simulink model "TosAeb"  (R2021a)
settings: Solver=FixedStepDiscrete  StartTime=0.0  StopTime=inf  FixedStep=0.01  RelTol=1e-3
undefined symbols (not defined in the file or in its folder): ACCModSts, AccSts1, AEB_ObjectMovingOrientation, AEB_ObjectMovingState, CN_BTCActive, CN_CurrentGearR, CN_CurrentGearR_DHT, CN_PTCActive, CN_VDCActive, CN_VSC_EspOff, CN_VSC_FCW_AEB_Error, CN_VSC_FCW_AEB_NoDispay, CN_VSC_FCW_AEB_PerfmDgdtn, CN_VSC_FCW_AEB_TempUnAvlbl, CN_VSC_VehStandstill, CNaccelAvoidMax, CNaccelAvoidMin, CNbrakePedalPress, CNcomfortStrLeftAccelMax, CNcomfortStrLeftAccelMin, CNcomfortStrRightAccelMax, CNcomfortStrRightAccelMin, CNDrvDoorOpen, CNDrvModeEco, CNDrvModeNorm, CNDrvModeOther, CNDrvModeSport, CNDrvSBRreminder, CNNull, CNobjInvalidID, CNPassDoorOpen, CNPassSBRreminder, CNPEBSAccelNegInf, CNPEBSAccelNull, CNPEBSTimeInf, CNPEBSTimeNull, CNSTOPMAX, CNSTOPNULL, CNsubjWidth, CNSwtOFF, CNSwtON, CNTTCMAX, CNTTCNULL, CNVehConfigAEBCycNone, CNVehConfigAEBNone, CNVehConfigAEBPedNone, CNVehConfigFCWNone, CNVehConfigJANone, CollisionSeverity, FALSE, FCWSnvty, JACycObjCalcInfoDefaultParams, JAPedObjCalcInfoDefaultParams, JAVehObjCalcInfoDefaultParams, latCycObjCalcInfoDefaultParams, latPedObjCalcInfoDefaultParams, longObjCalcInfoDefaultParams, ModSts, movingStatus, ObjectType ... (+1112)
libraries: simulink x3031, AEBSFunctionLib x50, Ta_Library x33, AEBSStateMachineLib x23, Mylibrary x12, simulink_extras x7, ObjSelect_lib x3, dspstat3 x2
(part 77 of 102)

== system TosAeb/FcwAebJa_Step_sys/ALG_AEBSystem/ALG_AEBController/AEB_JaObjCalcApp/JAVehTgtInfoCalc/AEBJAVehObjCalc/JAVehObjInfoCalc/ObjCrossFlgCal/CollisionProbCalc/Subsystem/ObjBoundingBoxfront/ObjRUCoordinate ==
  inputSignals : Inport
  Add : Sum  Inputs=+--  OutDataTypeStr=single
  Add1 : Sum  Inputs=+-+  OutDataTypeStr=single
  Bus Selector : BusSelector  OutputSignals=dxPreRefPointObj,ObjLengthCorr,sincosCoef.cosCoef,ObjWidthCorr,sincosCoef.sinCoef
  Bus Selector1 : BusSelector  OutputSignals=dyPreRefPointObj,ObjLengthCorr,sincosCoef.sinCoef,ObjWidthCorr,sincosCoef.cosCoef
  Gain : Gain  Gain=0.5  OutDataTypeStr=single
  Gain1 : Gain  Gain=0.5  OutDataTypeStr=single
  Product{,1..3} (4 alike) : Product  OutDataTypeStr=single
  dxPreRUObj : Outport
  dyPreRUObj : Outport  Port=2
  wires:
  inputSignals/1 -> Bus Selector1/1, Bus Selector/1
  Bus Selector/2 -> Product/1  "<ObjLengthCorr>"
  Bus Selector/3 -> Product/2  "<cosCoef>"
  Bus Selector/4 -> Product1/1  "<ObjWidthCorr>"
  Bus Selector/5 -> Product1/2  "<sinCoef>"
  Product1/1 -> Gain1/1
  Bus Selector/1 -> Add/1  "<dxPreRefPointObj>"
  Product/1 -> Add/2
  Add/1 -> dxPreRUObj/1
  Add1/1 -> dyPreRUObj/1
  Product2/1 -> Add1/2
  Product3/1 -> Gain/1
  Bus Selector1/2 -> Product2/1  "<ObjLengthCorr>"
  Bus Selector1/4 -> Product3/1  "<ObjWidthCorr>"
  Bus Selector1/3 -> Product2/2  "<sinCoef>"
  Bus Selector1/5 -> Product3/2  "<cosCoef>"
  Bus Selector1/1 -> Add1/1  "<dyPreRefPointObj>"
  Gain/1 -> Add1/3
  Gain1/1 -> Add/3

== system TosAeb/FcwAebJa_Step_sys/ALG_AEBSystem/ALG_AEBController/AEB_JaObjCalcApp/JAVehTgtInfoCalc/AEBJAVehObjCalc/JAVehObjInfoCalc/ObjCrossFlgCal/CollisionProbCalc/Subsystem/PreCrossFlgCalcFlow/VectorCrossAlgorithm/If Action Subsystem ==
  Action Port : ActionPort  ActionPortLabel=if(u1)
  Constant : Constant  Value=0  OutDataTypeStr=boolean
  CrossFlg : Outport
  wires:
  Constant/1 -> CrossFlg/1

== system TosAeb/FcwAebJa_Step_sys/ALG_AEBSystem/ALG_AEBController/AEB_JaObjCalcApp/JAVehTgtInfoCalc/AEBJAVehObjCalc/JAVehObjInfoCalc/ObjCrossFlgCal/CollisionProbCalc/Subsystem/PreCrossFlgCalcFlow/VectorCrossAlgorithm/If Action Subsystem1 ==
  vectorMulit : Inport
  Coordinations : Inport  Port=2
  Action Port : ActionPort  ActionPortLabel=else
  Bus Selector : BusSelector  OutputSignals=m2,m1,y1,n1,n2,x1
  Bus Selector1 : BusSelector  OutputSignals=x2,x1,y1,n1,y2,m1
  Subsystem : Subsystem
  t : Subsystem
  u : Subsystem
  CrossFlg : Outport
  wires:
  Coordinations/1 -> Bus Selector1/1, Bus Selector/1
  Bus Selector/2 -> t/2(m1)  "<m1>"
  Bus Selector/3 -> t/3(y1)  "<y1>"
  Bus Selector/1 -> t/1(m2)  "<m2>"
  Bus Selector/4 -> t/4(n1)  "<n1>"
  Bus Selector/5 -> t/5(n2)  "<n2>"
  Bus Selector/6 -> t/6(x1)  "<x1>"
  Bus Selector1/3 -> u/3(y1)  "<y1>"
  Bus Selector1/2 -> u/2(x1)  "<x1>"
  Bus Selector1/4 -> u/4(n1)  "<n1>"
  Bus Selector1/1 -> u/1(x2)  "<x2>"
  Bus Selector1/5 -> u/5(y2)  "<y2>"
  Bus Selector1/6 -> u/6(m1)  "<m1>"
  t/1(temp_t) -> Subsystem/1(temp_u)
  u/1(temp_u) -> Subsystem/2(temp_t)
  Subsystem/1(CrossFlg) -> CrossFlg/1
  vectorMulit/1 -> Subsystem/3(vectorMulit)

== system TosAeb/FcwAebJa_Step_sys/ALG_AEBSystem/ALG_AEBController/AEB_JaObjCalcApp/JAVehTgtInfoCalc/AEBJAVehObjCalc/JAVehObjInfoCalc/ObjCrossFlgCal/CollisionProbCalc/Subsystem/PreCrossFlgCalcFlow/VectorCrossAlgorithm/VectorMulit ==
  x1 : Inport  OutDataTypeStr=single
  x2 : Inport  Port=2  OutDataTypeStr=single
  n2 : Inport  Port=3  OutDataTypeStr=single
  n1 : Inport  Port=4  OutDataTypeStr=single
  y2 : Inport  Port=5  OutDataTypeStr=single
  y1 : Inport  Port=6  OutDataTypeStr=single
  m2 : Inport  Port=7  OutDataTypeStr=single
  m1 : Inport  Port=8  OutDataTypeStr=single
  Product : Product  OutDataTypeStr=single
  Product1 : Product  OutDataTypeStr=single
  Subtract{1..4} (4 alike) : Sum  Inputs=+-  OutDataTypeStr=single
  Subtract5 : Sum  Inputs=+-
  vectorMulit : Outport
  wires:
  x1/1 -> Subtract2/2
  x2/1 -> Subtract2/1
  Subtract2/1 -> Product/1
  Subtract3/1 -> Product/2
  y2/1 -> Subtract4/1
  y1/1 -> Subtract4/2
  m2/1 -> Subtract1/1
  m1/1 -> Subtract1/2
  Subtract4/1 -> Product1/1
  Subtract1/1 -> Product1/2
  Product/1 -> Subtract5/1
  Product1/1 -> Subtract5/2
  Subtract5/1 -> vectorMulit/1
  n2/1 -> Subtract3/1
  n1/1 -> Subtract3/2

== system TosAeb/FcwAebJa_Step_sys/ALG_AEBSystem/ALG_AEBController/AEB_JaObjCalcApp/JAVehTgtInfoCalc/AEBJAVehObjCalc/JAVehObjInfoCalc/ObjCrossFlgCal/CollisionProbCalc/Subsystem/SubjBoundingBox/SubjLLCoordinate ==
  inputSignals : Inport
  Add2 : Sum  Inputs=+--  OutDataTypeStr=single
  Add3 : Sum  Inputs=+-+  OutDataTypeStr=single
  Bus Selector2 : BusSelector  OutputSignals=dxPreRefPoint,dxRearBumperToRearAxis,sincosCoef.cosCoef,subjWidth,sincosCoef.sinCoef
  Bus Selector3 : BusSelector  OutputSignals=dyPreRefPoint,dxRearBumperToRearAxis,sincosCoef.sinCoef,subjWidth,sincosCoef.cosCoef
  Gain2 : Gain  Gain=0.5  OutDataTypeStr=single
  Gain3 : Gain  Gain=0.5  OutDataTypeStr=single
  Product{4..7} (4 alike) : Product  OutDataTypeStr=single
  dxPreLL : Outport
  dyPreLL : Outport  Port=2
  wires:
  Gain3/1 -> Add3/3
  Bus Selector3/3 -> Product6/2  "<sinCoef>"
  Add3/1 -> dyPreLL/1
  Product7/1 -> Gain3/1
  Product6/1 -> Add3/2
  Product4/1 -> Add2/2
  Bus Selector3/5 -> Product7/2  "<cosCoef>"
  Product5/1 -> Gain2/1
  Gain2/1 -> Add2/3
  Add2/1 -> dxPreLL/1
  Bus Selector3/4 -> Product7/1  "<subjWidth>"
  Bus Selector3/2 -> Product6/1  "<dxRearBumperToRearAxis>"
  Bus Selector3/1 -> Add3/1  "<dyPreRefPoint>"
  inputSignals/1 -> Bus Selector3/1, Bus Selector2/1
  Bus Selector2/4 -> Product5/1  "<subjWidth>"
  Bus Selector2/2 -> Product4/1  "<dxRearBumperToRearAxis>"
  Bus Selector2/3 -> Product4/2  "<cosCoef>"
  Bus Selector2/1 -> Add2/1  "<dxPreRefPoint>"
  Bus Selector2/5 -> Product5/2  "<sinCoef>"

== system TosAeb/FcwAebJa_Step_sys/ALG_AEBSystem/ALG_AEBController/AEB_JaObjCalcApp/JAVehTgtInfoCalc/AEBJAVehObjCalc/JAVehObjInfoCalc/ObjCrossFlgCal/CollisionProbCalc/Subsystem/SubjBoundingBox/SubjLUCoordinate ==
  inputSignals : Inport
  Add : Sum  Inputs=++-  OutDataTypeStr=single
  Add1 : Sum  Inputs=+++  OutDataTypeStr=single
  Bus Selector : BusSelector  OutputSignals=dxPreRefPoint,dxFrontBumperToRearAxis,sincosCoef.cosCoef,subjWidth,sincosCoef.sinCoef
  Bus Selector1 : BusSelector  OutputSignals=dyPreRefPoint,dxFrontBumperToRearAxis,sincosCoef.sinCoef,subjWidth,sincosCoef.cosCoef
  Gain : Gain  Gain=0.5  OutDataTypeStr=single
  Gain1 : Gain  Gain=0.5  OutDataTypeStr=single
  Product{,1..3} (4 alike) : Product  OutDataTypeStr=single
  dxPreLU : Outport
  dyPreLU : Outport  Port=2
  wires:
  Bus Selector/4 -> Product1/1  "<subjWidth>"
  Product3/1 -> Gain1/1
  Add/1 -> dxPreLU/1
  Bus Selector1/1 -> Add1/1  "<dyPreRefPoint>"
  Gain1/1 -> Add1/3
  Bus Selector1/4 -> Product3/1  "<subjWidth>"
  Product2/1 -> Add1/2
  Bus Selector/3 -> Product/2  "<cosCoef>"
  Add1/1 -> dyPreLU/1
  Bus Selector/5 -> Product1/2  "<sinCoef>"
  Bus Selector/2 -> Product/1  "<dxFrontBumperToRearAxis>"
  Bus Selector1/2 -> Product2/1  "<dxFrontBumperToRearAxis>"
  inputSignals/1 -> Bus Selector1/1, Bus Selector/1
  Bus Selector1/5 -> Product3/2  "<cosCoef>"
  Bus Selector/1 -> Add/1  "<dxPreRefPoint>"
  Gain/1 -> Add/3
  Product1/1 -> Gain/1
  Product/1 -> Add/2
  Bus Selector1/3 -> Product2/2  "<sinCoef>"

== system TosAeb/FcwAebJa_Step_sys/ALG_AEBSystem/ALG_AEBController/AEB_JaObjCalcApp/JAVehTgtInfoCalc/AEBJAVehObjCalc/JAVehObjInfoCalc/ObjCrossFlgCal/CollisionProbCalc/Subsystem/SubjBoundingBox/SubjRLCoordinate ==
  inputSignals : Inport
  Add6 : Sum  Inputs=+-+  OutDataTypeStr=single
  Add7 : Sum  Inputs=+--  OutDataTypeStr=single
  Bus Selector6 : BusSelector  OutputSignals=dxPreRefPoint,dxRearBumperToRearAxis,sincosCoef.cosCoef,subjWidth,sincosCoef.sinCoef
  Bus Selector7 : BusSelector  OutputSignals=dyPreRefPoint,dxRearBumperToRearAxis,sincosCoef.sinCoef,subjWidth,sincosCoef.cosCoef
  Gain6 : Gain  Gain=0.5  OutDataTypeStr=single
  Gain7 : Gain  Gain=0.5  OutDataTypeStr=single
  Product{12..15} (4 alike) : Product  OutDataTypeStr=single
  dxPreRL : Outport
  dyPreRL : Outport  Port=2
  wires:
  Product12/1 -> Add6/2
  Gain6/1 -> Add6/3
  Product13/1 -> Gain6/1
  Add6/1 -> dxPreRL/1
  Bus Selector6/4 -> Product13/1  "<subjWidth>"
  Bus Selector6/5 -> Product13/2  "<sinCoef>"
  Bus Selector6/1 -> Add6/1  "<dxPreRefPoint>"
  Bus Selector6/2 -> Product12/1  "<dxRearBumperToRearAxis>"
  Bus Selector6/3 -> Product12/2  "<cosCoef>"
  inputSignals/1 -> Bus Selector7/1, Bus Selector6/1
  Add7/1 -> dyPreRL/1
  Product14/1 -> Add7/2
  Gain7/1 -> Add7/3
  Bus Selector7/1 -> Add7/1  "<dyPreRefPoint>"
  Bus Selector7/2 -> Product14/1  "<dxRearBumperToRearAxis>"
  Bus Selector7/4 -> Product15/1  "<subjWidth>"
  Product15/1 -> Gain7/1
  Bus Selector7/5 -> Product15/2  "<cosCoef>"
  Bus Selector7/3 -> Product14/2  "<sinCoef>"

== system TosAeb/FcwAebJa_Step_sys/ALG_AEBSystem/ALG_AEBController/AEB_JaObjCalcApp/JAVehTgtInfoCalc/AEBJAVehObjCalc/JAVehObjInfoCalc/ObjCrossFlgCal/CollisionProbCalc/Subsystem/SubjBoundingBox/SubjRUCoordinate ==
  inputSignals : Inport
  Add4 : Sum  Inputs=+++  OutDataTypeStr=single
  Add5 : Sum  Inputs=++-  OutDataTypeStr=single
  Bus Selector4 : BusSelector  OutputSignals=dxPreRefPoint,dxFrontBumperToRearAxis,sincosCoef.cosCoef,subjWidth,sincosCoef.sinCoef
  Bus Selector5 : BusSelector  OutputSignals=dyPreRefPoint,dxFrontBumperToRearAxis,sincosCoef.sinCoef,subjWidth,sincosCoef.cosCoef
  Gain4 : Gain  Gain=0.5  OutDataTypeStr=single
  Gain5 : Gain  Gain=0.5  OutDataTypeStr=single
  Product{8..11} (4 alike) : Product  OutDataTypeStr=single
  dxPreRU : Outport
  dyPreRU : Outport  Port=2
  wires:
  Bus Selector4/4 -> Product9/1  "<subjWidth>"
  Bus Selector4/5 -> Product9/2  "<sinCoef>"
  Bus Selector4/3 -> Product8/2  "<cosCoef>"
  Bus Selector4/1 -> Add4/1  "<dxPreRefPoint>"
  inputSignals/1 -> Bus Selector5/1, Bus Selector4/1
  Bus Selector4/2 -> Product8/1  "<dxFrontBumperToRearAxis>"
  Product9/1 -> Gain4/1
  Bus Selector5/4 -> Product11/1  "<subjWidth>"
  Product10/1 -> Add5/2
  Product11/1 -> Gain5/1
  Add5/1 -> dyPreRU/1
  Gain5/1 -> Add5/3
  Bus Selector5/2 -> Product10/1  "<dxFrontBumperToRearAxis>"
  Bus Selector5/3 -> Product10/2  "<sinCoef>"
  Bus Selector5/5 -> Product11/2  "<cosCoef>"
  Add4/1 -> dxPreRU/1
  Gain4/1 -> Add4/3
  Product8/1 -> Add4/2
  Bus Selector5/1 -> Add5/1  "<dyPreRefPoint>"

== system TosAeb/FcwAebJa_Step_sys/ALG_AEBSystem/ALG_AEBController/AEB_JaObjCalcApp/JaCycTgtInfoCalc/JaCycObjCalcInfoCalc/JaCycObjInfoCalc/ObjCrossFlgCal/CollisionProbCalc/Subsystem/ObjBoundingBox/ObjLLCoordinate ==
  inputSignals : Inport
  Add : Sum  Inputs=+-  OutDataTypeStr=single
  Add1 : Sum  OutDataTypeStr=single
  Bus Selector : BusSelector  OutputSignals=dxPreRefPointObj,ObjWidthCorr,sincosCoef.sinCoef
  Bus Selector1 : BusSelector  OutputSignals=dyPreRefPointObj,ObjWidthCorr,sincosCoef.cosCoef
  Gain : Gain  Gain=0.5  OutDataTypeStr=single
  Gain1 : Gain  Gain=0.5  OutDataTypeStr=single
  Product1 : Product  OutDataTypeStr=single
  Product3 : Product  OutDataTypeStr=single
  dxPre_LLObj : Outport
  dyPreLLObj : Outport  Port=2
  wires:
  Bus Selector1/1 -> Add1/1  "<dyPreRefPointObj>"
  Bus Selector1/3 -> Product3/2  "<cosCoef>"
  Bus Selector1/2 -> Product3/1  "<ObjWidthCorr>"
  Product3/1 -> Gain/1
  Add1/1 -> dyPreLLObj/1
  Add/1 -> dxPre_LLObj/1
  Bus Selector/1 -> Add/1  "<dxPreRefPointObj>"
  Product1/1 -> Gain1/1
  Bus Selector/3 -> Product1/2  "<sinCoef>"
  Bus Selector/2 -> Product1/1  "<ObjWidthCorr>"
  inputSignals/1 -> Bus Selector1/1, Bus Selector/1
  Gain/1 -> Add1/2
  Gain1/1 -> Add/2

== system TosAeb/FcwAebJa_Step_sys/ALG_AEBSystem/ALG_AEBController/AEB_JaObjCalcApp/JaCycTgtInfoCalc/JaCycObjCalcInfoCalc/JaCycObjInfoCalc/ObjCrossFlgCal/CollisionProbCalc/Subsystem/ObjBoundingBox/ObjLUCoordinate ==
  inputSignals : Inport
  Add : Sum  Inputs=++-  OutDataTypeStr=single
  Add1 : Sum  Inputs=+++  OutDataTypeStr=single
  Bus Selector : BusSelector  OutputSignals=dxPreRefPointObj,ObjLengthCorr,sincosCoef.cosCoef,ObjWidthCorr,sincosCoef.sinCoef
  Bus Selector1 : BusSelector  OutputSignals=dyPreRefPointObj,ObjLengthCorr,sincosCoef.sinCoef,ObjWidthCorr,sincosCoef.cosCoef
  Gain : Gain  Gain=0.5  OutDataTypeStr=single
  Gain1 : Gain  Gain=0.5  OutDataTypeStr=single
  Product{,1..3} (4 alike) : Product  OutDataTypeStr=single
  dxPreLUObj : Outport
  dyPreLUobj : Outport  Port=2
  wires:
  inputSignals/1 -> Bus Selector1/1, Bus Selector/1
  Bus Selector/2 -> Product/1  "<ObjLengthCorr>"
  Bus Selector/3 -> Product/2  "<cosCoef>"
  Bus Selector/4 -> Product1/1  "<ObjWidthCorr>"
  Bus Selector/5 -> Product1/2  "<sinCoef>"
  Product1/1 -> Gain1/1
  Bus Selector/1 -> Add/1  "<dxPreRefPointObj>"
  Product/1 -> Add/2
  Add/1 -> dxPreLUObj/1
  Add1/1 -> dyPreLUobj/1
  Product2/1 -> Add1/2
  Product3/1 -> Gain/1
  Bus Selector1/2 -> Product2/1  "<ObjLengthCorr>"
  Bus Selector1/4 -> Product3/1  "<ObjWidthCorr>"
  Bus Selector1/3 -> Product2/2  "<sinCoef>"
  Bus Selector1/5 -> Product3/2  "<cosCoef>"
  Bus Selector1/1 -> Add1/1  "<dyPreRefPointObj>"
  Gain/1 -> Add1/3
  Gain1/1 -> Add/3

== system TosAeb/FcwAebJa_Step_sys/ALG_AEBSystem/ALG_AEBController/AEB_JaObjCalcApp/JaCycTgtInfoCalc/JaCycObjCalcInfoCalc/JaCycObjInfoCalc/ObjCrossFlgCal/CollisionProbCalc/Subsystem/ObjBoundingBox/ObjRLCoordinate ==
  inputSignals : Inport
  Add : Sum  OutDataTypeStr=single
  Add1 : Sum  Inputs=+-  OutDataTypeStr=single
  Bus Selector : BusSelector  OutputSignals=dxPreRefPointObj,ObjWidthCorr,sincosCoef.sinCoef
  Bus Selector1 : BusSelector  OutputSignals=dyPreRefPointObj,ObjWidthCorr,sincosCoef.cosCoef
  Gain : Gain  Gain=0.5  OutDataTypeStr=single
  Gain1 : Gain  Gain=0.5  OutDataTypeStr=single
  Product1 : Product  OutDataTypeStr=single
  Product3 : Product  OutDataTypeStr=single
  dxPreRLObj : Outport
  dyPreRLObj : Outport  Port=2
  wires:
  Bus Selector1/1 -> Add1/1  "<dyPreRefPointObj>"
  Bus Selector1/3 -> Product3/2  "<cosCoef>"
  Bus Selector1/2 -> Product3/1  "<ObjWidthCorr>"
  Product3/1 -> Gain1/1
  Add1/1 -> dyPreRLObj/1
  Add/1 -> dxPreRLObj/1
  Bus Selector/1 -> Add/1  "<dxPreRefPointObj>"
  Product1/1 -> Gain/1
  Bus Selector/3 -> Product1/2  "<sinCoef>"
  Bus Selector/2 -> Product1/1  "<ObjWidthCorr>"
  inputSignals/1 -> Bus Selector1/1, Bus Selector/1
  Gain/1 -> Add/2
  Gain1/1 -> Add1/2

== system TosAeb/FcwAebJa_Step_sys/ALG_AEBSystem/ALG_AEBController/AEB_JaObjCalcApp/JaCycTgtInfoCalc/JaCycObjCalcInfoCalc/JaCycObjInfoCalc/ObjCrossFlgCal/CollisionProbCalc/Subsystem/ObjBoundingBox/ObjRUCoordinate ==
  inputSignals : Inport
  Add : Sum  Inputs=+++  OutDataTypeStr=single
  Add1 : Sum  Inputs=++-  OutDataTypeStr=single
  Bus Selector : BusSelector  OutputSignals=dxPreRefPointObj,ObjLengthCorr,sincosCoef.cosCoef,ObjWidthCorr,sincosCoef.sinCoef
  Bus Selector1 : BusSelector  OutputSignals=dyPreRefPointObj,ObjLengthCorr,sincosCoef.sinCoef,ObjWidthCorr,sincosCoef.cosCoef
  Gain : Gain  Gain=0.5  OutDataTypeStr=single
  Gain1 : Gain  Gain=0.5  OutDataTypeStr=single
  Product{,1..3} (4 alike) : Product  OutDataTypeStr=single
  dxPreRUObj : Outport
  dyPreRUObj : Outport  Port=2
  wires:
  inputSignals/1 -> Bus Selector1/1, Bus Selector/1
  Bus Selector/2 -> Product/1  "<ObjLengthCorr>"
  Bus Selector/3 -> Product/2  "<cosCoef>"
  Bus Selector/4 -> Product1/1  "<ObjWidthCorr>"
  Bus Selector/5 -> Product1/2  "<sinCoef>"
  Product1/1 -> Gain1/1
  Bus Selector/1 -> Add/1  "<dxPreRefPointObj>"
  Product/1 -> Add/2
  Add/1 -> dxPreRUObj/1
  Add1/1 -> dyPreRUObj/1
  Product2/1 -> Add1/2
  Product3/1 -> Gain/1
  Bus Selector1/2 -> Product2/1  "<ObjLengthCorr>"
  Bus Selector1/4 -> Product3/1  "<ObjWidthCorr>"
  Bus Selector1/3 -> Product2/2  "<sinCoef>"
  Bus Selector1/5 -> Product3/2  "<cosCoef>"
  Bus Selector1/1 -> Add1/1  "<dyPreRefPointObj>"
  Gain/1 -> Add1/3
  Gain1/1 -> Add/3

== system TosAeb/FcwAebJa_Step_sys/ALG_AEBSystem/ALG_AEBController/AEB_JaObjCalcApp/JaCycTgtInfoCalc/JaCycObjCalcInfoCalc/JaCycObjInfoCalc/ObjCrossFlgCal/CollisionProbCalc/Subsystem/PreCrossFlgCalcFlow/VectorCrossAlgorithm/If Action Subsystem ==
  Action Port : ActionPort  ActionPortLabel=if(u1)
  Constant : Constant  Value=0  OutDataTypeStr=boolean
  CrossFlg : Outport
  wires:
  Constant/1 -> CrossFlg/1

== system TosAeb/FcwAebJa_Step_sys/ALG_AEBSystem/ALG_AEBController/AEB_JaObjCalcApp/JaCycTgtInfoCalc/JaCycObjCalcInfoCalc/JaCycObjInfoCalc/ObjCrossFlgCal/CollisionProbCalc/Subsystem/PreCrossFlgCalcFlow/VectorCrossAlgorithm/If Action Subsystem1 ==
  vectorMulit : Inport
  Coordinations : Inport  Port=2
  Action Port : ActionPort  ActionPortLabel=else
  Bus Selector : BusSelector  OutputSignals=m2,m1,y1,n1,n2,x1
  Bus Selector1 : BusSelector  OutputSignals=x2,x1,y1,n1,y2,m1
  Subsystem : Subsystem
  t : Subsystem
  u : Subsystem
  CrossFlg : Outport
  wires:
  Coordinations/1 -> Bus Selector1/1, Bus Selector/1
  Bus Selector/2 -> t/2(m1)  "<m1>"
  Bus Selector/3 -> t/3(y1)  "<y1>"
  Bus Selector/1 -> t/1(m2)  "<m2>"
  Bus Selector/4 -> t/4(n1)  "<n1>"
  Bus Selector/5 -> t/5(n2)  "<n2>"
  Bus Selector/6 -> t/6(x1)  "<x1>"
  Bus Selector1/3 -> u/3(y1)  "<y1>"
  Bus Selector1/2 -> u/2(x1)  "<x1>"
  Bus Selector1/4 -> u/4(n1)  "<n1>"
  Bus Selector1/1 -> u/1(x2)  "<x2>"
  Bus Selector1/5 -> u/5(y2)  "<y2>"
  Bus Selector1/6 -> u/6(m1)  "<m1>"
  t/1(temp_t) -> Subsystem/1(temp_u)
  u/1(temp_u) -> Subsystem/2(temp_t)
  Subsystem/1(CrossFlg) -> CrossFlg/1
  vectorMulit/1 -> Subsystem/3(vectorMulit)

== system TosAeb/FcwAebJa_Step_sys/ALG_AEBSystem/ALG_AEBController/AEB_JaObjCalcApp/JaCycTgtInfoCalc/JaCycObjCalcInfoCalc/JaCycObjInfoCalc/ObjCrossFlgCal/CollisionProbCalc/Subsystem/PreCrossFlgCalcFlow/VectorCrossAlgorithm/VectorMulit ==
  x1 : Inport  OutDataTypeStr=single
  x2 : Inport  Port=2  OutDataTypeStr=single
  n2 : Inport  Port=3  OutDataTypeStr=single
  n1 : Inport  Port=4  OutDataTypeStr=single
  y2 : Inport  Port=5  OutDataTypeStr=single
  y1 : Inport  Port=6  OutDataTypeStr=single
  m2 : Inport  Port=7  OutDataTypeStr=single
  m1 : Inport  Port=8  OutDataTypeStr=single
  Product : Product  OutDataTypeStr=single
  Product1 : Product  OutDataTypeStr=single
  Subtract{1..4} (4 alike) : Sum  Inputs=+-  OutDataTypeStr=single
  Subtract5 : Sum  Inputs=+-
  vectorMulit : Outport
  wires:
  x1/1 -> Subtract2/2
  x2/1 -> Subtract2/1
  Subtract2/1 -> Product/1
  Subtract3/1 -> Product/2
  y2/1 -> Subtract4/1
  y1/1 -> Subtract4/2
  m2/1 -> Subtract1/1
  m1/1 -> Subtract1/2
  Subtract4/1 -> Product1/1
  Subtract1/1 -> Product1/2
  Product/1 -> Subtract5/1
  Product1/1 -> Subtract5/2
  Subtract5/1 -> vectorMulit/1
  n2/1 -> Subtract3/1
  n1/1 -> Subtract3/2

== system TosAeb/FcwAebJa_Step_sys/ALG_AEBSystem/ALG_AEBController/AEB_JaObjCalcApp/JaCycTgtInfoCalc/JaCycObjCalcInfoCalc/JaCycObjInfoCalc/ObjCrossFlgCal/CollisionProbCalc/Subsystem/SubjBoundingBox/SubjLLCoordinate ==
  inputSignals : Inport
  Add2 : Sum  Inputs=+--  OutDataTypeStr=single
  Add3 : Sum  Inputs=+-+  OutDataTypeStr=single
  Bus Selector2 : BusSelector  OutputSignals=dxPreRefPoint,dxRearBumperToRearAxis,sincosCoef.cosCoef,subjWidth,sincosCoef.sinCoef
  Bus Selector3 : BusSelector  OutputSignals=dyPreRefPoint,dxRearBumperToRearAxis,sincosCoef.sinCoef,subjWidth,sincosCoef.cosCoef
  Gain2 : Gain  Gain=0.5  OutDataTypeStr=single
  Gain3 : Gain  Gain=0.5  OutDataTypeStr=single
  Product{4..7} (4 alike) : Product  OutDataTypeStr=single
  dxPreLL : Outport
  dyPreLL : Outport  Port=2
  wires:
  Gain3/1 -> Add3/3
  Bus Selector3/3 -> Product6/2  "<sinCoef>"
  Add3/1 -> dyPreLL/1
  Product7/1 -> Gain3/1
  Product6/1 -> Add3/2
  Product4/1 -> Add2/2
  Bus Selector3/5 -> Product7/2  "<cosCoef>"
  Product5/1 -> Gain2/1
  Gain2/1 -> Add2/3
  Add2/1 -> dxPreLL/1
  Bus Selector3/4 -> Product7/1  "<subjWidth>"
  Bus Selector3/2 -> Product6/1  "<dxRearBumperToRearAxis>"
  Bus Selector3/1 -> Add3/1  "<dyPreRefPoint>"
  inputSignals/1 -> Bus Selector3/1, Bus Selector2/1
  Bus Selector2/4 -> Product5/1  "<subjWidth>"
  Bus Selector2/2 -> Product4/1  "<dxRearBumperToRearAxis>"
  Bus Selector2/3 -> Product4/2  "<cosCoef>"
  Bus Selector2/1 -> Add2/1  "<dxPreRefPoint>"
  Bus Selector2/5 -> Product5/2  "<sinCoef>"

== system TosAeb/FcwAebJa_Step_sys/ALG_AEBSystem/ALG_AEBController/AEB_JaObjCalcApp/JaCycTgtInfoCalc/JaCycObjCalcInfoCalc/JaCycObjInfoCalc/ObjCrossFlgCal/CollisionProbCalc/Subsystem/SubjBoundingBox/SubjLUCoordinate ==
  inputSignals : Inport
  Add : Sum  Inputs=++-  OutDataTypeStr=single
  Add1 : Sum  Inputs=+++  OutDataTypeStr=single
  Bus Selector : BusSelector  OutputSignals=dxPreRefPoint,dxFrontBumperToRearAxis,sincosCoef.cosCoef,subjWidth,sincosCoef.sinCoef
  Bus Selector1 : BusSelector  OutputSignals=dyPreRefPoint,dxFrontBumperToRearAxis,sincosCoef.sinCoef,subjWidth,sincosCoef.cosCoef
  Gain : Gain  Gain=0.5  OutDataTypeStr=single
  Gain1 : Gain  Gain=0.5  OutDataTypeStr=single
  Product{,1..3} (4 alike) : Product  OutDataTypeStr=single
  dxPreLU : Outport
  dyPreLU : Outport  Port=2
  wires:
  Bus Selector/4 -> Product1/1  "<subjWidth>"
  Product3/1 -> Gain1/1
  Add/1 -> dxPreLU/1
  Bus Selector1/1 -> Add1/1  "<dyPreRefPoint>"
  Gain1/1 -> Add1/3
  Bus Selector1/4 -> Product3/1  "<subjWidth>"
  Product2/1 -> Add1/2
  Bus Selector/3 -> Product/2  "<cosCoef>"
  Add1/1 -> dyPreLU/1
  Bus Selector/5 -> Product1/2  "<sinCoef>"
  Bus Selector/2 -> Product/1  "<dxFrontBumperToRearAxis>"
  Bus Selector1/2 -> Product2/1  "<dxFrontBumperToRearAxis>"
  inputSignals/1 -> Bus Selector1/1, Bus Selector/1
  Bus Selector1/5 -> Product3/2  "<cosCoef>"
  Bus Selector/1 -> Add/1  "<dxPreRefPoint>"
  Gain/1 -> Add/3
  Product1/1 -> Gain/1
  Product/1 -> Add/2
  Bus Selector1/3 -> Product2/2  "<sinCoef>"

== system TosAeb/FcwAebJa_Step_sys/ALG_AEBSystem/ALG_AEBController/AEB_JaObjCalcApp/JaCycTgtInfoCalc/JaCycObjCalcInfoCalc/JaCycObjInfoCalc/ObjCrossFlgCal/CollisionProbCalc/Subsystem/SubjBoundingBox/SubjRLCoordinate ==
  inputSignals : Inport
  Add6 : Sum  Inputs=+-+  OutDataTypeStr=single
  Add7 : Sum  Inputs=+--  OutDataTypeStr=single
  Bus Selector6 : BusSelector  OutputSignals=dxPreRefPoint,dxRearBumperToRearAxis,sincosCoef.cosCoef,subjWidth,sincosCoef.sinCoef
  Bus Selector7 : BusSelector  OutputSignals=dyPreRefPoint,dxRearBumperToRearAxis,sincosCoef.sinCoef,subjWidth,sincosCoef.cosCoef
  Gain6 : Gain  Gain=0.5  OutDataTypeStr=single
  Gain7 : Gain  Gain=0.5  OutDataTypeStr=single
  Product{12..15} (4 alike) : Product  OutDataTypeStr=single
  dxPreRL : Outport
  dyPreRL : Outport  Port=2
  wires:
  Product12/1 -> Add6/2
  Gain6/1 -> Add6/3
  Product13/1 -> Gain6/1
  Add6/1 -> dxPreRL/1
  Bus Selector6/4 -> Product13/1  "<subjWidth>"
  Bus Selector6/5 -> Product13/2  "<sinCoef>"
  Bus Selector6/1 -> Add6/1  "<dxPreRefPoint>"
  Bus Selector6/2 -> Product12/1  "<dxRearBumperToRearAxis>"
  Bus Selector6/3 -> Product12/2  "<cosCoef>"
  inputSignals/1 -> Bus Selector7/1, Bus Selector6/1
  Add7/1 -> dyPreRL/1
  Product14/1 -> Add7/2
  Gain7/1 -> Add7/3
  Bus Selector7/1 -> Add7/1  "<dyPreRefPoint>"
  Bus Selector7/2 -> Product14/1  "<dxRearBumperToRearAxis>"
  Bus Selector7/4 -> Product15/1  "<subjWidth>"
  Product15/1 -> Gain7/1
  Bus Selector7/5 -> Product15/2  "<cosCoef>"
  Bus Selector7/3 -> Product14/2  "<sinCoef>"

== system TosAeb/FcwAebJa_Step_sys/ALG_AEBSystem/ALG_AEBController/AEB_JaObjCalcApp/JaCycTgtInfoCalc/JaCycObjCalcInfoCalc/JaCycObjInfoCalc/ObjCrossFlgCal/CollisionProbCalc/Subsystem/SubjBoundingBox/SubjRUCoordinate ==
  inputSignals : Inport
  Add4 : Sum  Inputs=+++  OutDataTypeStr=single
  Add5 : Sum  Inputs=++-  OutDataTypeStr=single
  Bus Selector4 : BusSelector  OutputSignals=dxPreRefPoint,dxFrontBumperToRearAxis,sincosCoef.cosCoef,subjWidth,sincosCoef.sinCoef
  Bus Selector5 : BusSelector  OutputSignals=dyPreRefPoint,dxFrontBumperToRearAxis,sincosCoef.sinCoef,subjWidth,sincosCoef.cosCoef
  Gain4 : Gain  Gain=0.5  OutDataTypeStr=single
  Gain5 : Gain  Gain=0.5  OutDataTypeStr=single
  Product{8..11} (4 alike) : Product  OutDataTypeStr=single
  dxPreRU : Outport
  dyPreRU : Outport  Port=2
  wires:
  Bus Selector4/4 -> Product9/1  "<subjWidth>"
  Bus Selector4/5 -> Product9/2  "<sinCoef>"
  Bus Selector4/3 -> Product8/2  "<cosCoef>"
  Bus Selector4/1 -> Add4/1  "<dxPreRefPoint>"
  inputSignals/1 -> Bus Selector5/1, Bus Selector4/1
  Bus Selector4/2 -> Product8/1  "<dxFrontBumperToRearAxis>"
  Product9/1 -> Gain4/1
  Bus Selector5/4 -> Product11/1  "<subjWidth>"
  Product10/1 -> Add5/2
  Product11/1 -> Gain5/1
  Add5/1 -> dyPreRU/1
  Gain5/1 -> Add5/3
  Bus Selector5/2 -> Product10/1  "<dxFrontBumperToRearAxis>"
  Bus Selector5/3 -> Product10/2  "<sinCoef>"
  Bus Selector5/5 -> Product11/2  "<cosCoef>"
  Add4/1 -> dxPreRU/1
  Gain4/1 -> Add4/3
  Product8/1 -> Add4/2
  Bus Selector5/1 -> Add5/1  "<dyPreRefPoint>"

== system TosAeb/FcwAebJa_Step_sys/ALG_AEBSystem/ALG_AEBController/AEB_LatObjInfoCalcApp/LatCycObjCalc/LatCycCollisonAssessInfoCalc/LatCycCollisonAssessInfoCalc/DistBrkCondCalc/CycDistBrakeFullCalc/DistPhase3Calc/tPhase3Calc/tEgoStopCalc ==
  subjVel : Inport
  subjLongAccelFilt : Inport  Port=2  OutDataTypeStr=single
  tJerkWork : Inport  Port=3  OutDataTypeStr=single
  Kjerk : Inport  Port=4  OutDataTypeStr=single
  accelMax : Inport  Port=5  OutDataTypeStr=single
  Add : Sum  Inputs=3  OutDataTypeStr=single
  Constant : Constant  Value=0.5  OutDataTypeStr=single
  Divide : Product  Inputs=*/  OutDataTypeStr=single
  Gain : Gain  Gain=-1  OutDataTypeStr=single
  Product : Product  Inputs=4  OutDataTypeStr=single
  Product1 : Product  OutDataTypeStr=single
  tEgoStop : Outport
  wires:
  Divide/1 -> Gain/1
  Product/1 -> Add/3
  Product1/1 -> Add/2
  tJerkWork/1 -> Product/2, Product/1, Product1/2
  Add/1 -> Divide/1
  Gain/1 -> tEgoStop/1
  accelMax/1 -> Divide/2
  Kjerk/1 -> Product/3
  subjLongAccelFilt/1 -> Product1/1
  Constant/1 -> Product/4
  subjVel/1 -> Add/1

== system TosAeb/FcwAebJa_Step_sys/ALG_AEBSystem/ALG_AEBController/AEB_LatObjInfoCalcApp/LatCycObjCalc/LatCycCollisonAssessInfoCalc/LatCycCollisonAssessInfoCalc/DistBrkCondCalc/CycDistBrakeFullCalc/DistPhase3Calc/tPhase3Calc/tPhase3Calc ==
  tVelDelta : Inport  OutDataTypeStr=single
  tEgoStop : Inport  Port=2  OutDataTypeStr=single
  MinMax : MinMax  Inputs=2  OutDataTypeStr=single
  tPhase3 : Outport
  wires:
  tVelDelta/1 -> MinMax/1
  tEgoStop/1 -> MinMax/2
  MinMax/1 -> tPhase3/1

== system TosAeb/FcwAebJa_Step_sys/ALG_AEBSystem/ALG_AEBController/AEB_LatObjInfoCalcApp/LatCycObjCalc/LatCycCollisonAssessInfoCalc/LatCycCollisonAssessInfoCalc/DistBrkCondCalc/CycDistBrakeFullCalc/DistPhase3Calc/tPhase3Calc/tVelDeltaCalc ==
  velDelta : Inport  OutDataTypeStr=single
  subjLongAccelFilt : Inport  Port=2  OutDataTypeStr=single
  tJerkWork : Inport  Port=3  OutDataTypeStr=single
  Kjerk : Inport  Port=4  OutDataTypeStr=single
  accelMax : Inport  Port=5  OutDataTypeStr=single
  Add : Sum  Inputs=+--  OutDataTypeStr=single
  Constant : Constant  Value=0.5  OutDataTypeStr=single
  Divide : Product  Inputs=*/  OutDataTypeStr=single
  Product : Product  Inputs=4  OutDataTypeStr=single
  Product1 : Product  OutDataTypeStr=single
  tVelDelta : Outport
  wires:
  Divide/1 -> tVelDelta/1
  accelMax/1 -> Divide/2
  Kjerk/1 -> Product/3
  velDelta/1 -> Add/1
  Add/1 -> Divide/1
  Product1/1 -> Add/2
  Constant/1 -> Product/4
  tJerkWork/1 -> Product/2, Product/1, Product1/2
  Product/1 -> Add/3
  subjLongAccelFilt/1 -> Product1/1

== system TosAeb/FcwAebJa_Step_sys/ALG_AEBSystem/ALG_AEBController/AEB_LatObjInfoCalcApp/LatCycObjCalc/LatCycCollisonAssessInfoCalc/LatCycCollisonAssessInfoCalc/DistBrkCondCalc/CycDistBrakePartCalc/DistPhase3Calc/tPhase3Calc/tEgoStopCalc ==
  subjVel : Inport
  subjLongAccelFilt : Inport  Port=2  OutDataTypeStr=single
  tJerkWork : Inport  Port=3  OutDataTypeStr=single
  Kjerk : Inport  Port=4  OutDataTypeStr=single
  accelMax : Inport  Port=5  OutDataTypeStr=single
  Add : Sum  Inputs=3  OutDataTypeStr=single
  Constant : Constant  Value=0.5  OutDataTypeStr=single
  Divide : Product  Inputs=*/  OutDataTypeStr=single
  Gain : Gain  Gain=-1  OutDataTypeStr=single
  Product : Product  Inputs=4  OutDataTypeStr=single
  Product1 : Product  OutDataTypeStr=single
  tEgoStop : Outport
  wires:
  subjVel/1 -> Add/1
  Constant/1 -> Product/4
  subjLongAccelFilt/1 -> Product1/1
  Kjerk/1 -> Product/3
  accelMax/1 -> Divide/2
  Gain/1 -> tEgoStop/1
  Add/1 -> Divide/1
  tJerkWork/1 -> Product1/2, Product/1, Product/2
  Product1/1 -> Add/2
  Product/1 -> Add/3
  Divide/1 -> Gain/1

== system TosAeb/FcwAebJa_Step_sys/ALG_AEBSystem/ALG_AEBController/AEB_LatObjInfoCalcApp/LatCycObjCalc/LatCycCollisonAssessInfoCalc/LatCycCollisonAssessInfoCalc/DistBrkCondCalc/CycDistBrakePartCalc/DistPhase3Calc/tPhase3Calc/tPhase3Calc ==
  tVelDelta : Inport  OutDataTypeStr=single
  tEgoStop : Inport  Port=2  OutDataTypeStr=single
  MinMax : MinMax  Inputs=2  OutDataTypeStr=single
  tPhase3 : Outport
  wires:
  MinMax/1 -> tPhase3/1
  tEgoStop/1 -> MinMax/2
  tVelDelta/1 -> MinMax/1

== system TosAeb/FcwAebJa_Step_sys/ALG_AEBSystem/ALG_AEBController/AEB_LatObjInfoCalcApp/LatCycObjCalc/LatCycCollisonAssessInfoCalc/LatCycCollisonAssessInfoCalc/DistBrkCondCalc/CycDistBrakePartCalc/DistPhase3Calc/tPhase3Calc/tVelDeltaCalc ==
  velDelta : Inport  OutDataTypeStr=single
  subjLongAccelFilt : Inport  Port=2  OutDataTypeStr=single
  tJerkWork : Inport  Port=3  OutDataTypeStr=single
  Kjerk : Inport  Port=4  OutDataTypeStr=single
  accelMax : Inport  Port=5  OutDataTypeStr=single
  Add : Sum  Inputs=+--  OutDataTypeStr=single
  Constant : Constant  Value=0.5  OutDataTypeStr=single
  Divide : Product  Inputs=*/  OutDataTypeStr=single
  Product : Product  Inputs=4  OutDataTypeStr=single
  Product1 : Product  OutDataTypeStr=single
  tVelDelta : Outport
  wires:
  subjLongAccelFilt/1 -> Product1/1
  Product/1 -> Add/3
  tJerkWork/1 -> Product1/2, Product/1, Product/2
  Constant/1 -> Product/4
  Product1/1 -> Add/2
  Add/1 -> Divide/1
  velDelta/1 -> Add/1
  Kjerk/1 -> Product/3
  accelMax/1 -> Divide/2
  Divide/1 -> tVelDelta/1

== system TosAeb/FcwAebJa_Step_sys/ALG_AEBSystem/ALG_AEBController/AEB_LatObjInfoCalcApp/LatCycObjCalc/LatCycCollisonAssessInfoCalc/LatCycCollisonAssessInfoCalc/DistBrkCondCalc/distBraking4FullBrakeCalc1/distPhase3Calc/distPhase3Calc/distPhase3Calc ==
  accelMax : Inport  OutDataTypeStr=single
  tWorkPhase3 : Inport  Port=2  OutDataTypeStr=single
  subjVelPhase2 : Inport  Port=3  OutDataTypeStr=single
  Add : Sum  OutDataTypeStr=single
  Gain : Gain  Gain=0.5  OutDataTypeStr=single
  Math Function : Math  Operator=square  SignedPower=on  OutDataTypeStr=single  SaturateOnIntegerOverflow=on
  Product : Product  OutDataTypeStr=single
  Product1 : Product  OutDataTypeStr=single
  distPhase3 : Outport
  wires:
  Add/1 -> distPhase3/1
  Product/1 -> Add/2
  Gain/1 -> Add/1
  Product1/1 -> Gain/1
  accelMax/1 -> Product1/1
  Math Function/1 -> Product1/2
  subjVelPhase2/1 -> Product/2
  tWorkPhase3/1 -> Math Function/1, Product/1

== system TosAeb/FcwAebJa_Step_sys/ALG_AEBSystem/ALG_AEBController/AEB_LatObjInfoCalcApp/LatCycObjCalc/LatCycCollisonAssessInfoCalc/LatCycCollisonAssessInfoCalc/DistBrkCondCalc/distBraking4FullBrakeCalc1/distPhase3Calc/distPhase3Calc/subjVelPhase2Calc ==
  note: 变更名称
  Kjerk : Inport  OutDataTypeStr=single
  tWorkPhase2 : Inport  Port=2  OutDataTypeStr=single
  accelWorkPhase1 : Inport  Port=3  OutDataTypeStr=single
  subjLongAccelCorr : Inport  Port=4  OutDataTypeStr=single
  tWorkPhase1 : Inport  Port=5  OutDataTypeStr=single
  Add : Sum  OutDataTypeStr=single
  Add2 : Sum  OutDataTypeStr=single
  Gain : Gain  Gain=0.5  OutDataTypeStr=single
  Gain1 : Gain  Gain=0.5  OutDataTypeStr=single
  Math Function : Math  Operator=square  SignedPower=on  OutDataTypeStr=single  SaturateOnIntegerOverflow=on
  Product : Product  OutDataTypeStr=single
  Product1 : Product  OutDataTypeStr=single
  Product2 : Product  OutDataTypeStr=single
  subjVelReducePhase2 : Outport
  subjVelPhase2 : Outport  Port=2
  wires:
  Gain1/1 -> subjVelPhase2/1
  subjLongAccelCorr/1 -> Product2/2
  Add2/1 -> Product2/1
  Product2/1 -> Gain1/1
  tWorkPhase2/1 -> Math Function/1, Product/1, Add2/1
  tWorkPhase1/1 -> Add2/2
  Add/1 -> subjVelReducePhase2/1
  Math Function/1 -> Product1/2
  Product/1 -> Add/2
  Product1/1 -> Gain/1
  Kjerk/1 -> Product1/1
  accelWorkPhase1/1 -> Product/2
  Gain/1 -> Add/1

== system TosAeb/FcwAebJa_Step_sys/ALG_AEBSystem/ALG_AEBController/AEB_LatObjInfoCalcApp/LatCycObjCalc/LatCycCollisonAssessInfoCalc/LatCycCollisonAssessInfoCalc/DistBrkCondCalc/distBraking4FullBrakeCalc1/distPhase3Calc/distPhase3Calc/tWorktimePhase3 ==
  note: 增加4输入接口
  velMaxReduce : Inport  OutDataTypeStr=single
  accelMax : Inport  Port=2  OutDataTypeStr=single
  subjVelReducePhase2 : Inport  Port=3  OutDataTypeStr=single
  subjVelPhase2 : Inport  Port=4  OutDataTypeStr=single
  Divide : Product  Inputs=*/  OutDataTypeStr=single
  Divide1 : Product  Inputs=*/  OutDataTypeStr=single
  MinMax : MinMax  Inputs=2  OutDataTypeStr=single
  Subtract : Sum  Inputs=+-  OutDataTypeStr=single
  tWorkPhase3 : Outport
  wires:
  MinMax/1 -> tWorkPhase3/1
  Divide1/1 -> MinMax/2
  Divide/1 -> MinMax/1
  [accelMax] -> Divide1/2
  Subtract/1 -> Divide1/1
  subjVelReducePhase2/1 -> Subtract/2
  accelMax/1 -> Divide/2, [accelMax]
  subjVelPhase2/1 -> Divide/1
  velMaxReduce/1 -> Subtract/1

== system TosAeb/FcwAebJa_Step_sys/ALG_AEBSystem/ALG_AEBController/AEB_LatObjInfoCalcApp/LatCycObjCalc/LatCycCollisonAssessInfoCalc/LatCycCollisonAssessInfoCalc/DistBrkCondCalc/distBraking4PartBrakeCalc1/distPhase3Calc/distPhase3Calc/distPhase3Calc ==
  accelMax : Inport  OutDataTypeStr=single
  tWorkPhase3 : Inport  Port=2  OutDataTypeStr=single
  subjVelPhase2 : Inport  Port=3  OutDataTypeStr=single
  Add : Sum  OutDataTypeStr=single
  Gain : Gain  Gain=0.5  OutDataTypeStr=single
  Math Function : Math  Operator=square  SignedPower=on  OutDataTypeStr=single  SaturateOnIntegerOverflow=on
  Product : Product  OutDataTypeStr=single
  Product1 : Product  OutDataTypeStr=single
  distPhase3 : Outport
  wires:
  tWorkPhase3/1 -> Product/1, Math Function/1
  subjVelPhase2/1 -> Product/2
  Math Function/1 -> Product1/2
  accelMax/1 -> Product1/1
  Product1/1 -> Gain/1
  Gain/1 -> Add/1
  Product/1 -> Add/2
  Add/1 -> distPhase3/1

== system TosAeb/FcwAebJa_Step_sys/ALG_AEBSystem/ALG_AEBController/AEB_LatObjInfoCalcApp/LatCycObjCalc/LatCycCollisonAssessInfoCalc/LatCycCollisonAssessInfoCalc/DistBrkCondCalc/distBraking4PartBrakeCalc1/distPhase3Calc/distPhase3Calc/subjVelPhase2Calc ==
  note: 变更名称
  Kjerk : Inport  OutDataTypeStr=single
  tWorkPhase2 : Inport  Port=2  OutDataTypeStr=single
  accelWorkPhase1 : Inport  Port=3  OutDataTypeStr=single
  subjLongAccelCorr : Inport  Port=4  OutDataTypeStr=single
  tWorkPhase1 : Inport  Port=5  OutDataTypeStr=single
  Add : Sum  OutDataTypeStr=single
  Add2 : Sum  OutDataTypeStr=single
  Gain : Gain  Gain=0.5  OutDataTypeStr=single
  Gain1 : Gain  Gain=0.5  OutDataTypeStr=single
  Math Function : Math  Operator=square  SignedPower=on  OutDataTypeStr=single  SaturateOnIntegerOverflow=on
  Product : Product  OutDataTypeStr=single
  Product1 : Product  OutDataTypeStr=single
  Product2 : Product  OutDataTypeStr=single
  subjVelReducePhase2 : Outport
  subjVelPhase2 : Outport  Port=2
  wires:
  Gain/1 -> Add/1
  accelWorkPhase1/1 -> Product/2
  Kjerk/1 -> Product1/1
  Product1/1 -> Gain/1
  Product/1 -> Add/2
  Math Function/1 -> Product1/2
  Add/1 -> subjVelReducePhase2/1
  tWorkPhase1/1 -> Add2/2
  tWorkPhase2/1 -> Add2/1, Product/1, Math Function/1
  Product2/1 -> Gain1/1
  Add2/1 -> Product2/1
  subjLongAccelCorr/1 -> Product2/2
  Gain1/1 -> subjVelPhase2/1

== system TosAeb/FcwAebJa_Step_sys/ALG_AEBSystem/ALG_AEBController/AEB_LatObjInfoCalcApp/LatCycObjCalc/LatCycCollisonAssessInfoCalc/LatCycCollisonAssessInfoCalc/DistBrkCondCalc/distBraking4PartBrakeCalc1/distPhase3Calc/distPhase3Calc/tWorktimePhase3 ==
  note: 增加4输入接口
  velMaxReduce : Inport  OutDataTypeStr=single
  accelMax : Inport  Port=2  OutDataTypeStr=single
  subjVelReducePhase2 : Inport  Port=3  OutDataTypeStr=single
  subjVelPhase2 : Inport  Port=4  OutDataTypeStr=single
  Divide : Product  Inputs=*/  OutDataTypeStr=single
  Divide1 : Product  Inputs=*/  OutDataTypeStr=single
  MinMax : MinMax  Inputs=2  OutDataTypeStr=single
  Subtract : Sum  Inputs=+-  OutDataTypeStr=single
  tWorkPhase3 : Outport
  wires:
  velMaxReduce/1 -> Subtract/1
  subjVelPhase2/1 -> Divide/1
  accelMax/1 -> [accelMax], Divide/2
  subjVelReducePhase2/1 -> Subtract/2
  Subtract/1 -> Divide1/1
  [accelMax] -> Divide1/2
  Divide/1 -> MinMax/1
  Divide1/1 -> MinMax/2
  MinMax/1 -> tWorkPhase3/1

== system TosAeb/FcwAebJa_Step_sys/ALG_AEBSystem/ALG_AEBController/AEB_LatObjInfoCalcApp/LatCycObjCalc/LatCycObjCalc/latCycObjInfoCalc/ObjColiisionRiskProbCalc/CollisionRiskProbCalc/ObjReginCorrCalc/AccelXYMaxMinCorrCalc/AccelXMaxMinCorrCalc ==
  vxAbsTargetCorr : Inport
  AccelMaxX : Inport  Port=2
  AccelMinX : Inport  Port=3
  Bus Creator : BusCreator  Inputs=2
  Bus Creator1 : BusCreator  Inputs=2
  Bus Creator2 : BusCreator  Inputs=2
  Display : Display
  Display2 : Display
  If : If  IfExpression=u1 < 0  ElseIfExpressions=u1 > 0
  Merge : Merge  Inputs=3
  ObjDwn2UpMoving : Subsystem  TreatAsAtomicUnit=on
  ObjStanding : Subsystem  TreatAsAtomicUnit=on
  ObjUp2DwnMoving : Subsystem  TreatAsAtomicUnit=on
  AccelXMaxMinCorr : Outport
  wires:
  Merge/1 -> AccelXMaxMinCorr/1  "AccelXMaxMinCorr"
  Bus Creator2/1 -> Merge/3
  Bus Creator/1 -> Merge/1
  Bus Creator1/1 -> Merge/2
  ObjStanding/2(AccelXMinCorr) -> Bus Creator2/2  "AccelXMinCorr"
  ObjStanding/1(AccelXMaxCorr) -> Bus Creator2/1  "AccelXMaxCorr"
  ObjDwn2UpMoving/2(AccelXMinCorr) -> Bus Creator1/2  "AccelXMinCorr"
  ObjDwn2UpMoving/1(AccelXMaxCorr) -> Bus Creator1/1  "AccelXMaxCorr"
  ObjUp2DwnMoving/2(AccelXMinCorr) -> Bus Creator/2  "AccelXMinCorr"
  ObjUp2DwnMoving/1(AccelXMaxCorr) -> Bus Creator/1  "AccelXMaxCorr"
  AccelMaxX/1 -> ObjDwn2UpMoving/1(AccelMaxX), ObjUp2DwnMoving/1(AccelMaxX)
  AccelMinX/1 -> Display2/1, [AccelMinX], ObjUp2DwnMoving/2(AccelMinX)
  [ObjStand] -> ObjStanding/Action
  [ObjDwn2Up] -> ObjDwn2UpMoving/Action
  If/3 -> [ObjStand]
  If/2 -> [ObjDwn2Up]
  If/1 -> ObjUp2DwnMoving/Action
  vxAbsTargetCorr/1 -> Display/1, If/1
  [AccelMinX] -> ObjDwn2UpMoving/2(AccelMinX)

== system TosAeb/FcwAebJa_Step_sys/ALG_AEBSystem/ALG_AEBController/AEB_LatObjInfoCalcApp/LatCycObjCalc/LatCycObjCalc/latCycObjInfoCalc/ObjColiisionRiskProbCalc/CollisionRiskProbCalc/ObjReginCorrCalc/AccelXYMaxMinCorrCalc/AccelXYMaxMinCalc ==
  maxAccelDecel : Inport
  sincosCoef : Inport  Port=2
  Abs : Abs
  Abs1 : Abs
  Bus Creator : BusCreator  Inputs=2
  Bus Selector : BusSelector  OutputSignals=signal1.maxAccel
  Bus Selector1 : BusSelector  OutputSignals=sincosCoef.cosCoef
  Bus Selector2 : BusSelector  OutputSignals=signal1.maxDecel
  Bus Selector3 : BusSelector  OutputSignals=sincosCoef.cosCoef
  Bus Selector4 : BusSelector  OutputSignals=signal1.maxAccel
  Bus Selector5 : BusSelector  OutputSignals=sincosCoef.sinCoef
  Bus Selector6 : BusSelector  OutputSignals=signal1.maxDecel
  Bus Selector7 : BusSelector  OutputSignals=sincosCoef.sinCoef
  Product{2,4..6} (4 alike) : Product
  AccelXMax : Outport
  AccelXMin : Outport  Port=2
  AccelYMax : Outport  Port=3
  AccelYMin : Outport  Port=4
  wires:
  Product2/1 -> AccelXMax/1  "AccelXMax"
  Product5/1 -> AccelYMax/1  "AccelYMax"
  Bus Selector6/1 -> Product6/1  "<maxDecel>"
  Bus Selector7/1 -> Abs/1  "<sinCoef>"
  Bus Creator/1 -> Bus Selector/1, Bus Selector1/1, Bus Selector2/1, Bus Selector3/1, Bus Selector4/1, Bus Selector5/1, Bus Selector6/1, Bus Selector7/1
  Product4/1 -> AccelXMin/1  "AccelXMin"
  Bus Selector2/1 -> Product4/1  "<maxDecel>"
  Bus Selector4/1 -> Product5/1  "<maxAccel>"
  Product6/1 -> AccelYMin/1  "AccelYMin"
  Bus Selector/1 -> Product2/1  "<maxAccel>"
  Bus Selector3/1 -> Product4/2  "<cosCoef>"
  Bus Selector1/1 -> Product2/2  "<cosCoef>"
  Bus Selector5/1 -> Abs1/1  "<sinCoef>"
  sincosCoef/1 -> Bus Creator/2
  maxAccelDecel/1 -> Bus Creator/1
  Abs/1 -> Product6/2
  Abs1/1 -> Product5/2

== system TosAeb/FcwAebJa_Step_sys/ALG_AEBSystem/ALG_AEBController/AEB_LatObjInfoCalcApp/LatCycObjCalc/LatCycObjCalc/latCycObjInfoCalc/ObjColiisionRiskProbCalc/CollisionRiskProbCalc/ObjReginCorrCalc/AccelXYMaxMinCorrCalc/AccelYMaxMinCorrCalc ==
  vyAbsTargetCorr : Inport
  AccelMaxY : Inport  Port=2
  AccelMinY : Inport  Port=3
  Bus Creator : BusCreator  Inputs=2
  Bus Creator1 : BusCreator  Inputs=2
  Bus Creator2 : BusCreator  Inputs=2
  If : If  IfExpression=u1 < 0  ElseIfExpressions=u1 > 0
  Merge : Merge  Inputs=3
  ObjLeft2RightMoving : Subsystem  TreatAsAtomicUnit=on
  ObjRight2LeftMoving : Subsystem  TreatAsAtomicUnit=on
  ObjStanding : Subsystem  TreatAsAtomicUnit=on
  AccelYMaxMinCorr : Outport
  wires:
  [AccelMinX] -> ObjRight2LeftMoving/2(AccelYMin)
  vyAbsTargetCorr/1 -> If/1
  If/1 -> ObjLeft2RightMoving/Action
  If/2 -> [ObjRight2Left]
  If/3 -> [ObjStand]
  [ObjRight2Left] -> ObjRight2LeftMoving/Action
  [ObjStand] -> ObjStanding/Action
  AccelMinY/1 -> ObjLeft2RightMoving/2(AccelYMin), [AccelMinX]
  AccelMaxY/1 -> ObjLeft2RightMoving/1(AccelYMax), ObjRight2LeftMoving/1(AccelYMax)
  ObjLeft2RightMoving/1(AccelYMaxCorr) -> Bus Creator/1  "AccelYMaxCorr"
  ObjLeft2RightMoving/2(AccelYMinCorr) -> Bus Creator/2  "AccelYMinCorr"
  ObjRight2LeftMoving/1(AccelYMaxCorr) -> Bus Creator1/1  "AccelYMaxCorr"
  ObjRight2LeftMoving/2(AccelYMinCorr) -> Bus Creator1/2  "AccelYMinCorr"
  ObjStanding/1(AccelYMaxCorr) -> Bus Creator2/1  "AccelYMaxCorr"
  ObjStanding/2(AccelYMinCorr) -> Bus Creator2/2  "AccelYMinCorr"
  Bus Creator1/1 -> Merge/2
  Bus Creator/1 -> Merge/1
  Bus Creator2/1 -> Merge/3
  Merge/1 -> AccelYMaxMinCorr/1  "AccelYMaxMinCorr"

== system TosAeb/FcwAebJa_Step_sys/ALG_AEBSystem/ALG_AEBController/AEB_LatObjInfoCalcApp/LatCycObjCalc/LatCycObjCalc/latCycObjInfoCalc/ObjColiisionRiskProbCalc/CollisionRiskProbCalc/ObjReginCorrCalc/AccelXYMaxMinCorrCalc/sincosCoefCalc ==
  angle : Inport
  Bus Creator : BusCreator  Inputs=2
  Sincos : Trigonometry  Operator=sincos
  sincosCoef : Outport
  wires:
  Bus Creator/1 -> sincosCoef/1  "sincosCoef"
  Sincos/2 -> Bus Creator/2  "cosCoef"
  angle/1 -> Sincos/1
  Sincos/1 -> Bus Creator/1  "sinCoef"

== system TosAeb/FcwAebJa_Step_sys/ALG_AEBSystem/ALG_AEBController/AEB_LatObjInfoCalcApp/LatCycObjCalc/LatCycObjCalc/latCycObjInfoCalc/ObjColiisionRiskProbCalc/CollisionRiskProbCalc/ObjReginCorrCalc/AccelXYMaxMinCorrCalc/vXYAngleCalc ==
  note: vy
      | vxAngle = atan(--------)
      | 				vx
  velAbsTargetCorr : Inport
  Bus Selector : BusSelector  OutputSignals=vyAbsObjCorr,vxAbsObjCorr
  Trigonometric Function : Trigonometry  Operator=atan2
  vXYAngle : Outport
  wires:
  Bus Selector/2 -> Trigonometric Function/2  "<vxAbsObjCorr>"
  Bus Selector/1 -> Trigonometric Function/1  "<vyAbsObjCorr>"
  Trigonometric Function/1 -> vXYAngle/1
  velAbsTargetCorr/1 -> Bus Selector/1

== system TosAeb/FcwAebJa_Step_sys/ALG_AEBSystem/ALG_AEBController/AEB_LatObjInfoCalcApp/LatCycObjCalc/LatCycObjCalc/latCycObjInfoCalc/ObjCrossFlgCal/CycPreCollisionCalcFunc/PreCrossFlgCalcFlow/VectorCrossAlgorithm/If Action Subsystem1/Subsystem ==
  temp_u : Inport  OutDataTypeStr=single
  temp_t : Inport  Port=2  OutDataTypeStr=single
  vectorMulit : Inport  Port=3
  Compare To Constant : simulink/Logic and Bit Operations/Compare To Constant  relop=>=  const=0  OutDataTypeStr=boolean
  Compare To Constant1 : simulink/Logic and Bit Operations/Compare To Constant  relop=<=  const=1  OutDataTypeStr=boolean
  Compare To Constant2 : simulink/Logic and Bit Operations/Compare To Constant  relop=>=  const=0  OutDataTypeStr=boolean
  Compare To Constant3 : simulink/Logic and Bit Operations/Compare To Constant  relop=<=  const=1  OutDataTypeStr=boolean
  Divide : Product  Inputs=*/
  Divide1 : Product  Inputs=*/
  Logical Operator : Logic  Inputs=4  AllPortsSameDT=off  OutDataTypeStr=boolean
  CrossFlg : Outport
  wires:
  Logical Operator/1 -> CrossFlg/1
  Compare To Constant3/1 -> Logical Operator/4
  Compare To Constant/1 -> Logical Operator/1
  Compare To Constant2/1 -> Logical Operator/3
  Compare To Constant1/1 -> Logical Operator/2
  Divide/1 -> Compare To Constant2/1, Compare To Constant3/1
  Divide1/1 -> Compare To Constant/1, Compare To Constant1/1
  temp_u/1 -> Divide1/1
  temp_t/1 -> Divide/1
  vectorMulit/1 -> Divide/2, Divide1/2

== system TosAeb/FcwAebJa_Step_sys/ALG_AEBSystem/ALG_AEBController/AEB_LatObjInfoCalcApp/LatCycObjCalc/LatCycObjCalc/latCycObjInfoCalc/ObjCrossFlgCal/CycPreCollisionCalcFunc/PreCrossFlgCalcFlow/VectorCrossAlgorithm/If Action Subsystem1/t ==
  m2 : Inport  OutDataTypeStr=single
  m1 : Inport  Port=2  OutDataTypeStr=single
  y1 : Inport  Port=3  OutDataTypeStr=single
  n1 : Inport  Port=4  OutDataTypeStr=single
  n2 : Inport  Port=5  OutDataTypeStr=single
  x1 : Inport  Port=6  OutDataTypeStr=single
  Product : Product  OutDataTypeStr=single
  Product1 : Product  OutDataTypeStr=single
  Subtract{1..5} (5 alike) : Sum  Inputs=+-  OutDataTypeStr=single
  temp_t : Outport
  wires:
  Subtract5/1 -> temp_t/1
  Product1/1 -> Subtract5/2
  Product/1 -> Subtract5/1
  Subtract1/1 -> Product1/2
  Subtract4/1 -> Product1/1
  x1/1 -> Subtract1/1
  n1/1 -> Subtract3/2, Subtract4/2
  n2/1 -> Subtract4/1
  Subtract3/1 -> Product/2
  Subtract2/1 -> Product/1
  y1/1 -> Subtract3/1
  m1/1 -> Subtract1/2, Subtract2/2
  m2/1 -> Subtract2/1

== system TosAeb/FcwAebJa_Step_sys/ALG_AEBSystem/ALG_AEBController/AEB_LatObjInfoCalcApp/LatCycObjCalc/LatCycObjCalc/latCycObjInfoCalc/ObjCrossFlgCal/CycPreCollisionCalcFunc/PreCrossFlgCalcFlow/VectorCrossAlgorithm/If Action Subsystem1/u ==
  x2 : Inport  OutDataTypeStr=single
  x1 : Inport  Port=2  OutDataTypeStr=single
  y1 : Inport  Port=3  OutDataTypeStr=single
  n1 : Inport  Port=4  OutDataTypeStr=single
  y2 : Inport  Port=5  OutDataTypeStr=single
  m1 : Inport  Port=6  OutDataTypeStr=single
  Product : Product  OutDataTypeStr=single
  Product1 : Product  OutDataTypeStr=single
  Subtract{1..5} (5 alike) : Sum  Inputs=+-  OutDataTypeStr=single
  temp_u : Outport
  wires:
  Subtract5/1 -> temp_u/1
  m1/1 -> Subtract1/2
  x2/1 -> Subtract2/1
  x1/1 -> Subtract2/2, Subtract1/1
  y1/1 -> Subtract3/1, Subtract4/2
  Subtract2/1 -> Product/1
  Subtract3/1 -> Product/2
  y2/1 -> Subtract4/1
  n1/1 -> Subtract3/2
  Subtract4/1 -> Product1/1
  Subtract1/1 -> Product1/2
  Product/1 -> Subtract5/1
  Product1/1 -> Subtract5/2

== system TosAeb/FcwAebJa_Step_sys/ALG_AEBSystem/ALG_AEBController/AEB_LatObjInfoCalcApp/LatPedObjCalc/LatPedCollisonAssessInfo/LatPedCollisonAssessInfo/LatPedLongDistCondCalc/PedDistBrakeFullCalc/DistPhase3Calc/tPhase3Calc/tEgoStopCalc ==
  subjVel : Inport
  subjLongAccelFilt : Inport  Port=2  OutDataTypeStr=single
  tJerkWork : Inport  Port=3  OutDataTypeStr=single
  Kjerk : Inport  Port=4  OutDataTypeStr=single
  accelMax : Inport  Port=5  OutDataTypeStr=single
  Add : Sum  Inputs=3  OutDataTypeStr=single
  Constant : Constant  Value=0.5  OutDataTypeStr=single
  Divide : Product  Inputs=*/  OutDataTypeStr=single
  Gain : Gain  Gain=-1  OutDataTypeStr=single
  Product : Product  Inputs=4  OutDataTypeStr=single
  Product1 : Product  OutDataTypeStr=single
  tEgoStop : Outport
  wires:
  Divide/1 -> Gain/1
  Product/1 -> Add/3
  Product1/1 -> Add/2
  tJerkWork/1 -> Product/2, Product/1, Product1/2
  Add/1 -> Divide/1
  Gain/1 -> tEgoStop/1
  accelMax/1 -> Divide/2
  Kjerk/1 -> Product/3
  subjLongAccelFilt/1 -> Product1/1
  Constant/1 -> Product/4
  subjVel/1 -> Add/1

== system TosAeb/FcwAebJa_Step_sys/ALG_AEBSystem/ALG_AEBController/AEB_LatObjInfoCalcApp/LatPedObjCalc/LatPedCollisonAssessInfo/LatPedCollisonAssessInfo/LatPedLongDistCondCalc/PedDistBrakeFullCalc/DistPhase3Calc/tPhase3Calc/tPhase3Calc ==
  tVelDelta : Inport  OutDataTypeStr=single
  tEgoStop : Inport  Port=2  OutDataTypeStr=single
  MinMax : MinMax  Inputs=2  OutDataTypeStr=single
  tPhase3 : Outport
  wires:
  tVelDelta/1 -> MinMax/1
  tEgoStop/1 -> MinMax/2
  MinMax/1 -> tPhase3/1
